simulink model "AuxClimaCtlMgr"  (R2022b)
settings: Solver=FixedStepDiscrete  StartTime=0.0  StopTime=10.0  FixedStep=0.2  RelTol=1e-3
(part 2 of 2)

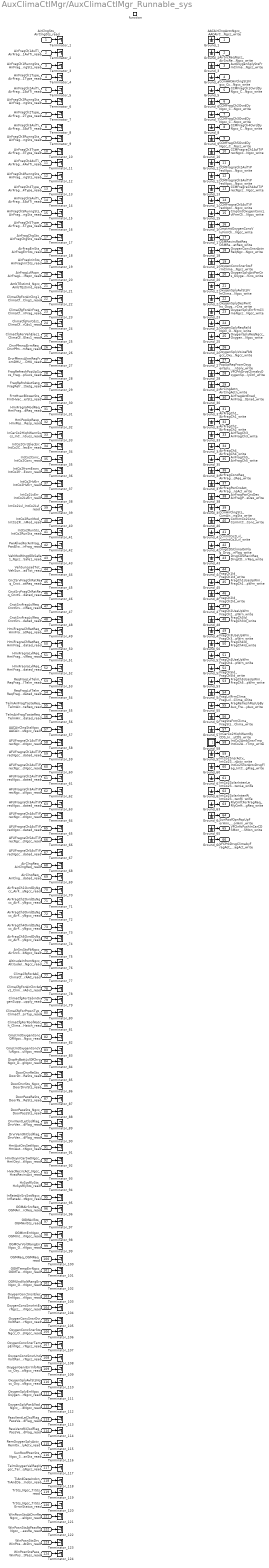
== system AuxClimaCtlMgr/AuxClimaCtlMgr_Runnable_sys ==
  AirClngSts_AirClngSts_read : Inport
  AirFragCh1AvlTi_AirFragCh1AvlTi_read : Inport  Port=2
  AirFragCh1RunngSts_AirFragCh1RunngSts_read : Inport  Port=3
  AirFragCh1Type_AirFragCh1Type_read : Inport  Port=4
  AirFragCh2AvlTi_AirFragCh2AvlTi_read : Inport  Port=5
  AirFragCh2RunngSts_AirFragCh2RunngSts_read : Inport  Port=6
  AirFragCh2Type_AirFragCh2Type_read : Inport  Port=7
  AirFragCh3AvlTi_AirFragCh3AvlTi_read : Inport  Port=8
  AirFragCh3RunngSts_AirFragCh3RunngSts_read : Inport  Port=9
  AirFragCh3Type_AirFragCh3Type_read : Inport  Port=10
  AirFragCh4AvlTi_AirFragCh4AvlTi_read : Inport  Port=11
  AirFragCh4RunngSts_AirFragCh4RunngSts_read : Inport  Port=12
  AirFragCh4Type_AirFragCh4Type_read : Inport  Port=13
  AirFragCh5AvlTi_AirFragCh5AvlTi_read : Inport  Port=14
  AirFragCh5RunngSts_AirFragCh5RunngSts_read : Inport  Port=15
  AirFragCh5Type_AirFragCh5Type_read : Inport  Port=16
  AirFragChgSts_AirFragChgSts_read : Inport  Port=17
  AirFragErrSts_AirFragErrSts_read : Inport  Port=18
  AirFragInitSts_AirFragInitSts_read : Inport  Port=19
  AirFragLvlRspn_AirFragLvlRspn_read : Inport  Port=20
  AmbTEstimd_Ngcc_AmbTEstimd_read : Inport  Port=21
  ClimaCfgForAirClng1_ClimaCfgForAirClng1_read : Inport  Port=22
  ClimaCfgForAirFrag_ClimaCfgForAirFrag_read : Inport  Port=23
  ClimaCfgForCds1_ClimaCfgForCds1_read : Inport  Port=24
  ClimaCfgForVehElec1_ClimaCfgForVehElec1_read : Inport  Port=25
  DrvrPfmncAlrmReq_DrvrPfmncAlrmReq_read : Inport  Port=26
  DrvrPfmncAlrmReqFromDHU_DrvrPfmncAlrmReqFromDHU_read : Inport  Port=27
  FragRefreshPopUpSucs_FragRefreshPopUpSucs_read : Inport  Port=28
  FragRefrshAutSetg_FragRefrshAutSetg_read : Inport  Port=29
  FrntHvacBlowerSts_FrntHvacBlowerSts_read : Inport  Port=30
  HmiFragraModReq_HmiFragraModReq_read : Inport  Port=31
  HmiPopUpResp_HmiPopUpResp_read : Inport  Port=32
  InCarCo2HighWarnSucs_InCarCo2HighWarnSucs_read : Inport  Port=33
  IntCo2CircElecErr_IntCo2CircElecErr_read : Inport  Port=34
  IntCo2Conc_IntCo2Conc_read : Inport  Port=35
  IntCo2fromExsrc_IntCo2fromExsrc_read : Inport  Port=36
  IntCo2HvErr_IntCo2HvErr_read : Inport  Port=37
  IntCo2LvErr_IntCo2LvErr_read : Inport  Port=38
  IntCo2Lvl_IntCo2Lvl_read : Inport  Port=39
  IntCo2RunMod_IntCo2RunMod_read : Inport  Port=40
  IntCo2RunSts_IntCo2RunSts_read : Inport  Port=41
  PwrAllwdForAirFrag_PwrAllwdForAirFrag_read : Inport  Port=42
  VehModMngtGlbSafe1_Ngcc_VehModMngtGlbSafe1_read : Inport  Port=43
  VehSunLoadTot_VehSunLoadTot_read : Inport  Port=44
  CnctSrvFragChRatReq_CnctSrvFragChRatReq_read : Inport  Port=45
  CnctSrvFragChRatReq_CnctSrvFragChRatReq_IsUpdated_read : Inport  Port=46
  CnctSrvFragLvlReq_CnctSrvFragLvlReq_read : Inport  Port=47
  CnctSrvFragLvlReq_CnctSrvFragLvlReq_IsUpdated_read : Inport  Port=48
  HmiFragraChRatReq_HmiFragraChRatReq_read : Inport  Port=49
  HmiFragraChRatReq_HmiFragraChRatReq_IsUpdated_read : Inport  Port=50
  HmiFragraLvlReq_HmiFragraLvlReq_read : Inport  Port=51
  HmiFragraLvlReq_HmiFragraLvlReq_IsUpdated_read : Inport  Port=52
  ReqFragLvlTelm_ReqFragLvlTelm_read : Inport  Port=53
  ReqFragLvlTelm_ReqFragLvlTelm_IsUpdated_read : Inport  Port=54
  TelmAirFragTasteReq_TelmAirFragTasteReq_read : Inport  Port=55
  TelmAirFragTasteReq_TelmAirFragTasteReq_IsUpdated_read : Inport  Port=56
  AACAirClngStsNgcc_AACAirClngStsNgcc_read : Inport  Port=57
  AFUFragraCh1AvlTiPrecNgcc_AFUFragraCh1AvlTiPrecNgcc_read : Inport  Port=58
  AFUFragraCh1AvlTiPrecNgcc_AFUFragraCh1AvlTiPrecNgcc_IsUpdated_read : Inport  Port=59
  AFUFragraCh2AvlTiPrecNgcc_AFUFragraCh2AvlTiPrecNgcc_read : Inport  Port=60
  AFUFragraCh2AvlTiPrecNgcc_AFUFragraCh2AvlTiPrecNgcc_IsUpdated_read : Inport  Port=61
  AFUFragraCh3AvlTiPrecNgcc_AFUFragraCh3AvlTiPrecNgcc_read : Inport  Port=62
  AFUFragraCh3AvlTiPrecNgcc_AFUFragraCh3AvlTiPrecNgcc_IsUpdated_read : Inport  Port=63
  AFUFragraCh4AvlTiPrecNgcc_AFUFragraCh4AvlTiPrecNgcc_read : Inport  Port=64
  AFUFragraCh4AvlTiPrecNgcc_AFUFragraCh4AvlTiPrecNgcc_IsUpdated_read : Inport  Port=65
  AFUFragraCh5AvlTiPrecNgcc_AFUFragraCh5AvlTiPrecNgcc_read : Inport  Port=66
  AFUFragraCh5AvlTiPrecNgcc_AFUFragraCh5AvlTiPrecNgcc_IsUpdated_read : Inport  Port=67
  AirClngReq_AirClngReq_read : Inport  Port=68
  AirClngReq_AirClngReq_IsUpdated_read : Inport  Port=69
  AirFragCh1OvrdDyNgcc_AirFragCh1OvrdDyNgcc_read : Inport  Port=70
  AirFragCh2OvrdDyNgcc_AirFragCh2OvrdDyNgcc_read : Inport  Port=71
  AirFragCh3OvrdDyNgcc_AirFragCh3OvrdDyNgcc_read : Inport  Port=72
  AirFragCh4OvrdDyNgcc_AirFragCh4OvrdDyNgcc_read : Inport  Port=73
  AirFragCh5OvrdDyNgcc_AirFragCh5OvrdDyNgcc_read : Inport  Port=74
  AirSrcStsFbNgcc_AirSrcStsFbNgcc_read : Inport  Port=75
  AltitudeInfromNgcc_AltitudeInfromNgcc_read : Inport  Port=76
  ClimaCfgForAAC_ClimaCfgForAAC_read : Inport  Port=77
  ClimaCfgForAirClnrAdv1_ClimaCfgForAirClnrAdv1_read : Inport  Port=78
  ClimaCfgForCabinOxgenSupply_ClimaCfgForCabinOxgenSupply_read : Inport  Port=79
  ClimaCfgForPrpsnTyp_ClimaCfgForPrpsnTyp_read : Inport  Port=80
  ClimaCfgForRoofHatch_ClimaCfgForRoofHatch_read : Inport  Port=81
  CmptmtOxygenConcQFNgcc_CmptmtOxygenConcQFNgcc_read : Inport  Port=82
  CmptmtOxygenConcVluNgcc_CmptmtOxygenConcVluNgcc_read : Inport  Port=83
  DispHvBattLvlOfChrgNgcc_DispHvBattLvlOfChrgNgcc_read : Inport  Port=84
  DoorDrvrReSts_DoorDrvrReSts_read : Inport  Port=85
  DoorDrvrSts_Ngcc_DoorDrvrSts_read : Inport  Port=86
  DoorPassReSts_DoorPassReSts_read : Inport  Port=87
  DoorPassSts_Ngcc_DoorPassSts_read : Inport  Port=88
  DrvrVentLeClsdFlag_DrvrVentLeClsdFlag_read : Inport  Port=89
  DrvrVentRiClsdFlag_DrvrVentRiClsdFlag_read : Inport  Port=90
  HmiAutOxySetNgcc_HmiAutOxySetNgcc_read : Inport  Port=91
  HmiOxyinCarSwtNgcc_HmiOxyinCarSwtNgcc_read : Inport  Port=92
  HvacRecircAct_Ngcc_HvacRecircAct_read : Inport  Port=93
  HvSysRlySts_HvSysRlySts_read : Inport  Port=94
  InflateAirSrcSwtNgcc_InflateAirSrcSwtNgcc_read : Inport  Port=95
  OGMAirSrcReq_OGMAirSrcReq_read : Inport  Port=96
  OGMAvlSts_OGMAvlSts_read : Inport  Port=97
  OGMIntErrNgcc_OGMIntErrNgcc_read : Inport  Port=98
  OGMOvrVoltRangErrNgcc_OGMOvrVoltRangErrNgcc_read : Inport  Port=99
  OGMReq_OGMReq_read : Inport  Port=100
  OGMTempErrNgcc_OGMTempErrNgcc_read : Inport  Port=101
  OGMUndVoltRangErrNgcc_OGMUndVoltRangErrNgcc_read : Inport  Port=102
  OxygenConcSnsrElecErrNgcc_OxygenConcSnsrElecErrNgcc_read : Inport  Port=103
  OxygenConcSnsrIntErrNgcc_OxygenConcSnsrIntErrNgcc_read : Inport  Port=104
  OxygenConcSnsrOvrVoltRangErrNgcc_OxygenConcSnsrOvrVoltRangErrNgcc_read : Inport  Port=105
  OxygenConcSnsrStsNgcc_OxygenConcSnsrStsNgcc_read : Inport  Port=106
  OxygenConcSnsrTempErrNgcc_OxygenConcSnsrTempErrNgcc_read : Inport  Port=107
  OxygenConcSnsrUndVoltRangErrNgcc_OxygenConcSnsrUndVoltRangErrNgcc_read : Inport  Port=108
  OxygenGenrErrInfoNgcc_OxygenGenrErrInfoNgcc_read : Inport  Port=109
  OxygenSplyAvlStsNgcc_OxygenSplyAvlStsNgcc_read : Inport  Port=110
  OxygenSplyErrNgcc_OxygenSplyErrNgcc_read : Inport  Port=111
  OxygenSplyPwrAllwdNgcc_OxygenSplyPwrAllwdNgcc_read : Inport  Port=112
  PassVentLeClsdFlag_PassVentLeClsdFlag_read : Inport  Port=113
  PassVentRiClsdFlag_PassVentRiClsdFlag_read : Inport  Port=114
  RemOxygenSplyActv_RemOxygenSplyActv_read : Inport  Port=115
  SunRoofPosnSts_Ngcc_SunRoofPosnSts_read : Inport  Port=116
  TelmOxygenValReqNgcc_TelmOxygenValReqNgcc_read : Inport  Port=117
  TiAndDateIndcn_TiAndDateIndcn_read : Inport  Port=118
  TrSts_Ngcc_TrSts_read : Inport  Port=119
  TrSts_Ngcc_TrSts_ErrorStatus_read : Inport  Port=120
  WinPosnStsAtDrvrReNgcc_WinPosnStsAtDrvrReNgcc_read : Inport  Port=121
  WinPosnStsAtPassReNgcc_WinPosnStsAtPassRe_read : Inport  Port=122
  WinPosnStsDrv_WinPosnStsAtDrv_read : Inport  Port=123
  WinPosnStsPass_WinPosnStsAtPass_read : Inport  Port=124
  function : TriggerPort  TriggerType=function-call  VariantControl=(inherit)  SampleTimeType=periodic  SampleTime=-1
  Ground_{1..57,59..66} (65 alike) : Ground
  Ground_58 : Subsystem
  Terminator_{1..124} (124 alike) : Terminator
  AACAirClngActnNgcc_AACAirClngActnNgcc_write : Outport
      settings: EnsureOutportIsVirtual=on
  AirSrcReqNgcc_AirSrcReqNgcc_write : Outport  Port=2
      settings: EnsureOutportIsVirtual=on
  AutOxygenSplyStsFrmClimaNgcc_AutOxygenSplyStsFrmClimaNgcc_write : Outport  Port=3
      settings: EnsureOutportIsVirtual=on
  CcmAACAirClngStsNgcc_CcmAACAirClngStsNgcc_write : Outport  Port=4
      settings: EnsureOutportIsVirtual=on
  CCMFragCh1OvrdDyNgcc_CCMFragCh1OvrdDyNgcc_write : Outport  Port=5
      settings: EnsureOutportIsVirtual=on
  CCMFragCh2OvrdDyNgcc_CCMFragCh2OvrdDyNgcc_write : Outport  Port=6
      settings: EnsureOutportIsVirtual=on
  CCMFragCh3OvrdDyNgcc_CCMFragCh3OvrdDyNgcc_write : Outport  Port=7
      settings: EnsureOutportIsVirtual=on
  CCMFragCh4OvrdDyNgcc_CCMFragCh4OvrdDyNgcc_write : Outport  Port=8
      settings: EnsureOutportIsVirtual=on
  CCMFragCh5OvrdDyNgcc_CCMFragCh5OvrdDyNgcc_write : Outport  Port=9
      settings: EnsureOutportIsVirtual=on
  CCMFragraCh1AvlTiPrecNgcc_CCMFragraCh1AvlTiPrecNgcc_write : Outport  Port=10
      settings: EnsureOutportIsVirtual=on
  CCMFragraCh2AvlTiPrecNgcc_CCMFragraCh2AvlTiPrecNgcc_write : Outport  Port=11
      settings: EnsureOutportIsVirtual=on
  CCMFragraCh3AvlTiPrecNgcc_CCMFragraCh3AvlTiPrecNgcc_write : Outport  Port=12
      settings: EnsureOutportIsVirtual=on
  CCMFragraCh4AvlTiPrecNgcc_CCMFragraCh4AvlTiPrecNgcc_write : Outport  Port=13
      settings: EnsureOutportIsVirtual=on
  CCMFragraCh5AvlTiPrecNgcc_CCMFragraCh5AvlTiPrecNgcc_write : Outport  Port=14
      settings: EnsureOutportIsVirtual=on
  CmptmtOxygenConcLvlFrmClimaNgcc_CmptmtOxygenConcLvlFrmClimaNgcc_write : Outport  Port=15
      settings: EnsureOutportIsVirtual=on
  CmptmtOxygenConcVluFrmClimaNgcc_CmptmtOxygenConcVluFrmClimaNgcc_write : Outport  Port=16
      settings: EnsureOutportIsVirtual=on
  OSMRecircRatReq_OSMRecircRatReq_write : Outport  Port=17
      settings: EnsureOutportIsVirtual=on
  OxygenConcSnsrActnReqNgcc_OxygenConcSnsrActnReqNgcc_write : Outport  Port=18
      settings: EnsureOutportIsVirtual=on
  OxygenConcSnsrStsFrmClimaNgcc_OxygenConcSnsrStsFrmClimaNgcc_write : Outport  Port=19
      settings: EnsureOutportIsVirtual=on
  OxygenSplyActPwrCns_OxygenSplyActPwrCns_write : Outport  Port=20
      settings: EnsureOutportIsVirtual=on
  OxygenSplyAvlStsFrmClimaNgcc_OxygenSplyAvlStsFrmClimaNgcc_write : Outport  Port=21
      settings: EnsureOutportIsVirtual=on
  OxygenSplyDesPwrCns_OxygenSplyDesPwrCns_write : Outport  Port=22
      settings: EnsureOutportIsVirtual=on
  OxygenSplyErrFrmClimaNgcc_OxygenSplyErrFrmClimaNgcc_write : Outport  Port=23
      settings: EnsureOutportIsVirtual=on
  OxygenSplyReqFaildNgcc_OxygenSplyReqFaildNgcc_write : Outport  Port=24
      settings: EnsureOutportIsVirtual=on
  OxygenSplyReqNgcc_OxygenSplyReqNgcc_write : Outport  Port=25
      settings: EnsureOutportIsVirtual=on
  OxygenSplyVoiceFbNgcc_OxygenSplyVoiceFbNgcc_write : Outport  Port=26
      settings: EnsureOutportIsVirtual=on
  PopUpReqFromOxygenSply_PopUpReqFromOxygenSply_write : Outport  Port=27
      settings: EnsureOutportIsVirtual=on
  VFCPrkDrvgClimabyOxygenSplyCtrl_VFCPrkDrvgClimabyOxygenSplyCtrl_write : Outport  Port=28
      settings: EnsureOutportIsVirtual=on
  AirClngActn_AirClngActn_write : Outport  Port=29
      settings: EnsureOutportIsVirtual=on
  AirFragActEnad_AirFragActEnad_write : Outport  Port=30
      settings: EnsureOutportIsVirtual=on
  AirFragCh1_AirFragCh1_write : Outport  Port=31
      settings: EnsureOutportIsVirtual=on
  AirFragCh2_AirFragCh2_write : Outport  Port=32
      settings: EnsureOutportIsVirtual=on
  AirFragCh3_AirFragCh3_write : Outport  Port=33
      settings: EnsureOutportIsVirtual=on
  AirFragCh4_AirFragCh4_write : Outport  Port=34
      settings: EnsureOutportIsVirtual=on
  AirFragCh5_AirFragCh5_write : Outport  Port=35
      settings: EnsureOutportIsVirtual=on
  AirFragConcReq_AirFragConcReq_write : Outport  Port=36
      settings: EnsureOutportIsVirtual=on
  AirFragPwrCnsAct_AirFragPwrCnsAct_write : Outport  Port=37
      settings: EnsureOutportIsVirtual=on
  AirFragPwrCnsDes_AirFragPwrCnsDes_write : Outport  Port=38
      settings: EnsureOutportIsVirtual=on
  CcmAirClngSts_CcmAirClngSts_write : Outport  Port=39
      settings: EnsureOutportIsVirtual=on
  CcmIntCo2Conc_CcmIntCo2Conc_write : Outport  Port=40
      settings: EnsureOutportIsVirtual=on
  CcmIntCo2Lvl_CcmIntCo2Lvl_write : Outport  Port=41
      settings: EnsureOutportIsVirtual=on
  DrvgCDSClimaOnFlag_DrvgCDSClimaOnFlag_write : Outport  Port=42
      settings: EnsureOutportIsVirtual=on
  DrvgCDSRecirReq_DrvgCDSRecirReq_write : Outport  Port=43
      settings: EnsureOutportIsVirtual=on
  FragCh1Id_FragCh1Id_write : Outport  Port=44
      settings: EnsureOutportIsVirtual=on
  FragCh1UseUpWrn_FragCh1UseUpWrn_write : Outport  Port=45
      settings: EnsureOutportIsVirtual=on
  FragCh2Id_FragCh2Id_write : Outport  Port=46
      settings: EnsureOutportIsVirtual=on
  FragCh2UseUpWrn_FragCh2UseUpWrn_write : Outport  Port=47
      settings: EnsureOutportIsVirtual=on
  FragCh3Id_FragCh3Id_write : Outport  Port=48
      settings: EnsureOutportIsVirtual=on
  FragCh3UseUpWrn_FragCh3UseUpWrn_write : Outport  Port=49
      settings: EnsureOutportIsVirtual=on
  FragCh4Id_FragCh4Id_write : Outport  Port=50
      settings: EnsureOutportIsVirtual=on
  FragCh4UseUpWrn_FragCh4UseUpWrn_write : Outport  Port=51
      settings: EnsureOutportIsVirtual=on
  FragCh5Id_FragCh5Id_write : Outport  Port=52
      settings: EnsureOutportIsVirtual=on
  FragCh5UseUpWrn_FragCh5UseUpWrn_write : Outport  Port=53
      settings: EnsureOutportIsVirtual=on
  FragLvlFrmClima_FragLvlFrmClima_write : Outport  Port=54
      settings: EnsureOutportIsVirtual=on
  FragRefreshPopUpByAux_FragRefreshPopUpByAux_write : Outport  Port=55
      settings: EnsureOutportIsVirtual=on
  FragStsFrmClima_FragStsFrmClima_write : Outport  Port=56
      settings: EnsureOutportIsVirtual=on
  InCarCo2HighWarnByCDS_InCarCo2HighWarnByCDS_write : Outport  Port=57
      settings: EnsureOutportIsVirtual=on
  IntCo2AmbSnsrTmp_IntCo2AmbSnsrTmp_write : Outport  Port=58
      settings: EnsureOutportIsVirtual=on
  IntCo2SnsrActv_IntCo2SnsrActv_write : Outport  Port=59
      settings: EnsureOutportIsVirtual=on
  IntCo2SnsrActvDrvgFlag_IntCo2SnsrActvDrvgFlag_write : Outport  Port=60
      settings: EnsureOutportIsVirtual=on
  IntCo2SolarIntenLe_IntCo2SolarIntenLe_write : Outport  Port=61
      settings: EnsureOutportIsVirtual=on
  IntCo2SolarIntenRi_IntCo2SolarIntenRi_write : Outport  Port=62
      settings: EnsureOutportIsVirtual=on
  RlyCmftForFragReq_RlyCmftForFragReq_write : Outport  Port=63
      settings: EnsureOutportIsVirtual=on
  SunRoofOpnPopUpForHmi_SunRoofOpnPopUpForHmi_write : Outport  Port=64
      settings: EnsureOutportIsVirtual=on
  VFCInfoPushInCarCDSMon_VFCInfoPushInCarCDSMon_write : Outport  Port=65
      settings: EnsureOutportIsVirtual=on
  VFCPrkDrvgClimabyFragAct_VFCPrkDrvgClimabyFragAct_write : Outport  Port=66
      settings: EnsureOutportIsVirtual=on
  wires:
  AirClngSts_AirClngSts_read/1 -> Terminator_1/1
  AirFragCh1AvlTi_AirFragCh1AvlTi_read/1 -> Terminator_2/1
  AirFragCh1RunngSts_AirFragCh1RunngSts_read/1 -> Terminator_3/1
  AirFragCh1Type_AirFragCh1Type_read/1 -> Terminator_4/1
  AirFragCh2AvlTi_AirFragCh2AvlTi_read/1 -> Terminator_5/1
  AirFragCh2RunngSts_AirFragCh2RunngSts_read/1 -> Terminator_6/1
  AirFragCh2Type_AirFragCh2Type_read/1 -> Terminator_7/1
  AirFragCh3AvlTi_AirFragCh3AvlTi_read/1 -> Terminator_8/1
  AirFragCh3RunngSts_AirFragCh3RunngSts_read/1 -> Terminator_9/1
  AirFragCh3Type_AirFragCh3Type_read/1 -> Terminator_10/1
  AirFragCh4AvlTi_AirFragCh4AvlTi_read/1 -> Terminator_11/1
  AirFragCh4RunngSts_AirFragCh4RunngSts_read/1 -> Terminator_12/1
  AirFragCh4Type_AirFragCh4Type_read/1 -> Terminator_13/1
  AirFragCh5AvlTi_AirFragCh5AvlTi_read/1 -> Terminator_14/1
  AirFragCh5RunngSts_AirFragCh5RunngSts_read/1 -> Terminator_15/1
  AirFragCh5Type_AirFragCh5Type_read/1 -> Terminator_16/1
  AirFragChgSts_AirFragChgSts_read/1 -> Terminator_17/1
  AirFragErrSts_AirFragErrSts_read/1 -> Terminator_18/1
  AirFragInitSts_AirFragInitSts_read/1 -> Terminator_19/1
  AirFragLvlRspn_AirFragLvlRspn_read/1 -> Terminator_20/1
  AmbTEstimd_Ngcc_AmbTEstimd_read/1 -> Terminator_21/1
  ClimaCfgForAirClng1_ClimaCfgForAirClng1_read/1 -> Terminator_22/1
  ClimaCfgForAirFrag_ClimaCfgForAirFrag_read/1 -> Terminator_23/1
  ClimaCfgForCds1_ClimaCfgForCds1_read/1 -> Terminator_24/1
  ClimaCfgForVehElec1_ClimaCfgForVehElec1_read/1 -> Terminator_25/1
  DrvrPfmncAlrmReq_DrvrPfmncAlrmReq_read/1 -> Terminator_26/1
  DrvrPfmncAlrmReqFromDHU_DrvrPfmncAlrmReqFromDHU_read/1 -> Terminator_27/1
  FragRefreshPopUpSucs_FragRefreshPopUpSucs_read/1 -> Terminator_28/1
  FragRefrshAutSetg_FragRefrshAutSetg_read/1 -> Terminator_29/1
  FrntHvacBlowerSts_FrntHvacBlowerSts_read/1 -> Terminator_30/1
  HmiFragraModReq_HmiFragraModReq_read/1 -> Terminator_31/1
  HmiPopUpResp_HmiPopUpResp_read/1 -> Terminator_32/1
  InCarCo2HighWarnSucs_InCarCo2HighWarnSucs_read/1 -> Terminator_33/1
  IntCo2CircElecErr_IntCo2CircElecErr_read/1 -> Terminator_34/1
  IntCo2Conc_IntCo2Conc_read/1 -> Terminator_35/1
  IntCo2fromExsrc_IntCo2fromExsrc_read/1 -> Terminator_36/1
  IntCo2HvErr_IntCo2HvErr_read/1 -> Terminator_37/1
  IntCo2LvErr_IntCo2LvErr_read/1 -> Terminator_38/1
  IntCo2Lvl_IntCo2Lvl_read/1 -> Terminator_39/1
  IntCo2RunMod_IntCo2RunMod_read/1 -> Terminator_40/1
  IntCo2RunSts_IntCo2RunSts_read/1 -> Terminator_41/1
  PwrAllwdForAirFrag_PwrAllwdForAirFrag_read/1 -> Terminator_42/1
  VehModMngtGlbSafe1_Ngcc_VehModMngtGlbSafe1_read/1 -> Terminator_43/1
  VehSunLoadTot_VehSunLoadTot_read/1 -> Terminator_44/1
  CnctSrvFragChRatReq_CnctSrvFragChRatReq_read/1 -> Terminator_45/1
  CnctSrvFragChRatReq_CnctSrvFragChRatReq_IsUpdated_read/1 -> Terminator_46/1
  CnctSrvFragLvlReq_CnctSrvFragLvlReq_read/1 -> Terminator_47/1
  CnctSrvFragLvlReq_CnctSrvFragLvlReq_IsUpdated_read/1 -> Terminator_48/1
  HmiFragraChRatReq_HmiFragraChRatReq_read/1 -> Terminator_49/1
  HmiFragraChRatReq_HmiFragraChRatReq_IsUpdated_read/1 -> Terminator_50/1
  HmiFragraLvlReq_HmiFragraLvlReq_read/1 -> Terminator_51/1
  HmiFragraLvlReq_HmiFragraLvlReq_IsUpdated_read/1 -> Terminator_52/1
  ReqFragLvlTelm_ReqFragLvlTelm_read/1 -> Terminator_53/1
  ReqFragLvlTelm_ReqFragLvlTelm_IsUpdated_read/1 -> Terminator_54/1
  TelmAirFragTasteReq_TelmAirFragTasteReq_read/1 -> Terminator_55/1
  TelmAirFragTasteReq_TelmAirFragTasteReq_IsUpdated_read/1 -> Terminator_56/1
  AACAirClngStsNgcc_AACAirClngStsNgcc_read/1 -> Terminator_57/1
  AFUFragraCh1AvlTiPrecNgcc_AFUFragraCh1AvlTiPrecNgcc_read/1 -> Terminator_58/1
  AFUFragraCh1AvlTiPrecNgcc_AFUFragraCh1AvlTiPrecNgcc_IsUpdated_read/1 -> Terminator_59/1
  AFUFragraCh2AvlTiPrecNgcc_AFUFragraCh2AvlTiPrecNgcc_read/1 -> Terminator_60/1
  AFUFragraCh2AvlTiPrecNgcc_AFUFragraCh2AvlTiPrecNgcc_IsUpdated_read/1 -> Terminator_61/1
  AFUFragraCh3AvlTiPrecNgcc_AFUFragraCh3AvlTiPrecNgcc_read/1 -> Terminator_62/1
  AFUFragraCh3AvlTiPrecNgcc_AFUFragraCh3AvlTiPrecNgcc_IsUpdated_read/1 -> Terminator_63/1
  AFUFragraCh4AvlTiPrecNgcc_AFUFragraCh4AvlTiPrecNgcc_read/1 -> Terminator_64/1
  AFUFragraCh4AvlTiPrecNgcc_AFUFragraCh4AvlTiPrecNgcc_IsUpdated_read/1 -> Terminator_65/1
  AFUFragraCh5AvlTiPrecNgcc_AFUFragraCh5AvlTiPrecNgcc_read/1 -> Terminator_66/1
  AFUFragraCh5AvlTiPrecNgcc_AFUFragraCh5AvlTiPrecNgcc_IsUpdated_read/1 -> Terminator_67/1
  AirClngReq_AirClngReq_read/1 -> Terminator_68/1
  AirClngReq_AirClngReq_IsUpdated_read/1 -> Terminator_69/1
  AirFragCh1OvrdDyNgcc_AirFragCh1OvrdDyNgcc_read/1 -> Terminator_70/1
  AirFragCh2OvrdDyNgcc_AirFragCh2OvrdDyNgcc_read/1 -> Terminator_71/1
  AirFragCh3OvrdDyNgcc_AirFragCh3OvrdDyNgcc_read/1 -> Terminator_72/1
  AirFragCh4OvrdDyNgcc_AirFragCh4OvrdDyNgcc_read/1 -> Terminator_73/1
  AirFragCh5OvrdDyNgcc_AirFragCh5OvrdDyNgcc_read/1 -> Terminator_74/1
  AirSrcStsFbNgcc_AirSrcStsFbNgcc_read/1 -> Terminator_75/1
  AltitudeInfromNgcc_AltitudeInfromNgcc_read/1 -> Terminator_76/1
  ClimaCfgForAAC_ClimaCfgForAAC_read/1 -> Terminator_77/1
  ClimaCfgForAirClnrAdv1_ClimaCfgForAirClnrAdv1_read/1 -> Terminator_78/1
  ClimaCfgForCabinOxgenSupply_ClimaCfgForCabinOxgenSupply_read/1 -> Terminator_79/1
  ClimaCfgForPrpsnTyp_ClimaCfgForPrpsnTyp_read/1 -> Terminator_80/1
  ClimaCfgForRoofHatch_ClimaCfgForRoofHatch_read/1 -> Terminator_81/1
  CmptmtOxygenConcQFNgcc_CmptmtOxygenConcQFNgcc_read/1 -> Terminator_82/1
  CmptmtOxygenConcVluNgcc_CmptmtOxygenConcVluNgcc_read/1 -> Terminator_83/1
  DispHvBattLvlOfChrgNgcc_DispHvBattLvlOfChrgNgcc_read/1 -> Terminator_84/1
  DoorDrvrReSts_DoorDrvrReSts_read/1 -> Terminator_85/1
  DoorDrvrSts_Ngcc_DoorDrvrSts_read/1 -> Terminator_86/1
  DoorPassReSts_DoorPassReSts_read/1 -> Terminator_87/1
  DoorPassSts_Ngcc_DoorPassSts_read/1 -> Terminator_88/1
  DrvrVentLeClsdFlag_DrvrVentLeClsdFlag_read/1 -> Terminator_89/1
  DrvrVentRiClsdFlag_DrvrVentRiClsdFlag_read/1 -> Terminator_90/1
  HmiAutOxySetNgcc_HmiAutOxySetNgcc_read/1 -> Terminator_91/1
  HmiOxyinCarSwtNgcc_HmiOxyinCarSwtNgcc_read/1 -> Terminator_92/1
  HvacRecircAct_Ngcc_HvacRecircAct_read/1 -> Terminator_93/1
  HvSysRlySts_HvSysRlySts_read/1 -> Terminator_94/1
  InflateAirSrcSwtNgcc_InflateAirSrcSwtNgcc_read/1 -> Terminator_95/1
  OGMAirSrcReq_OGMAirSrcReq_read/1 -> Terminator_96/1
  OGMAvlSts_OGMAvlSts_read/1 -> Terminator_97/1
  OGMIntErrNgcc_OGMIntErrNgcc_read/1 -> Terminator_98/1
  OGMOvrVoltRangErrNgcc_OGMOvrVoltRangErrNgcc_read/1 -> Terminator_99/1
  OGMReq_OGMReq_read/1 -> Terminator_100/1
  OGMTempErrNgcc_OGMTempErrNgcc_read/1 -> Terminator_101/1
  OGMUndVoltRangErrNgcc_OGMUndVoltRangErrNgcc_read/1 -> Terminator_102/1
  OxygenConcSnsrElecErrNgcc_OxygenConcSnsrElecErrNgcc_read/1 -> Terminator_103/1
  OxygenConcSnsrIntErrNgcc_OxygenConcSnsrIntErrNgcc_read/1 -> Terminator_104/1
  OxygenConcSnsrOvrVoltRangErrNgcc_OxygenConcSnsrOvrVoltRangErrNgcc_read/1 -> Terminator_105/1
  OxygenConcSnsrStsNgcc_OxygenConcSnsrStsNgcc_read/1 -> Terminator_106/1
  OxygenConcSnsrTempErrNgcc_OxygenConcSnsrTempErrNgcc_read/1 -> Terminator_107/1
  OxygenConcSnsrUndVoltRangErrNgcc_OxygenConcSnsrUndVoltRangErrNgcc_read/1 -> Terminator_108/1
  OxygenGenrErrInfoNgcc_OxygenGenrErrInfoNgcc_read/1 -> Terminator_109/1
  OxygenSplyAvlStsNgcc_OxygenSplyAvlStsNgcc_read/1 -> Terminator_110/1
  OxygenSplyErrNgcc_OxygenSplyErrNgcc_read/1 -> Terminator_111/1
  OxygenSplyPwrAllwdNgcc_OxygenSplyPwrAllwdNgcc_read/1 -> Terminator_112/1
  PassVentLeClsdFlag_PassVentLeClsdFlag_read/1 -> Terminator_113/1
  PassVentRiClsdFlag_PassVentRiClsdFlag_read/1 -> Terminator_114/1
  RemOxygenSplyActv_RemOxygenSplyActv_read/1 -> Terminator_115/1
  SunRoofPosnSts_Ngcc_SunRoofPosnSts_read/1 -> Terminator_116/1
  TelmOxygenValReqNgcc_TelmOxygenValReqNgcc_read/1 -> Terminator_117/1
  TiAndDateIndcn_TiAndDateIndcn_read/1 -> Terminator_118/1
  TrSts_Ngcc_TrSts_read/1 -> Terminator_119/1
  TrSts_Ngcc_TrSts_ErrorStatus_read/1 -> Terminator_120/1
  WinPosnStsAtDrvrReNgcc_WinPosnStsAtDrvrReNgcc_read/1 -> Terminator_121/1
  WinPosnStsAtPassReNgcc_WinPosnStsAtPassRe_read/1 -> Terminator_122/1
  WinPosnStsDrv_WinPosnStsAtDrv_read/1 -> Terminator_123/1
  WinPosnStsPass_WinPosnStsAtPass_read/1 -> Terminator_124/1
  Ground_1/1 -> AACAirClngActnNgcc_AACAirClngActnNgcc_write/1
  Ground_2/1 -> AirSrcReqNgcc_AirSrcReqNgcc_write/1
  Ground_3/1 -> AutOxygenSplyStsFrmClimaNgcc_AutOxygenSplyStsFrmClimaNgcc_write/1
  Ground_4/1 -> CcmAACAirClngStsNgcc_CcmAACAirClngStsNgcc_write/1
  Ground_5/1 -> CCMFragCh1OvrdDyNgcc_CCMFragCh1OvrdDyNgcc_write/1
  Ground_6/1 -> CCMFragCh2OvrdDyNgcc_CCMFragCh2OvrdDyNgcc_write/1
  Ground_7/1 -> CCMFragCh3OvrdDyNgcc_CCMFragCh3OvrdDyNgcc_write/1
  Ground_8/1 -> CCMFragCh4OvrdDyNgcc_CCMFragCh4OvrdDyNgcc_write/1
  Ground_9/1 -> CCMFragCh5OvrdDyNgcc_CCMFragCh5OvrdDyNgcc_write/1
  Ground_10/1 -> CCMFragraCh1AvlTiPrecNgcc_CCMFragraCh1AvlTiPrecNgcc_write/1
  Ground_11/1 -> CCMFragraCh2AvlTiPrecNgcc_CCMFragraCh2AvlTiPrecNgcc_write/1
  Ground_12/1 -> CCMFragraCh3AvlTiPrecNgcc_CCMFragraCh3AvlTiPrecNgcc_write/1
  Ground_13/1 -> CCMFragraCh4AvlTiPrecNgcc_CCMFragraCh4AvlTiPrecNgcc_write/1
  Ground_14/1 -> CCMFragraCh5AvlTiPrecNgcc_CCMFragraCh5AvlTiPrecNgcc_write/1
  Ground_15/1 -> CmptmtOxygenConcLvlFrmClimaNgcc_CmptmtOxygenConcLvlFrmClimaNgcc_write/1
  Ground_16/1 -> CmptmtOxygenConcVluFrmClimaNgcc_CmptmtOxygenConcVluFrmClimaNgcc_write/1
  Ground_17/1 -> OSMRecircRatReq_OSMRecircRatReq_write/1
  Ground_18/1 -> OxygenConcSnsrActnReqNgcc_OxygenConcSnsrActnReqNgcc_write/1
  Ground_19/1 -> OxygenConcSnsrStsFrmClimaNgcc_OxygenConcSnsrStsFrmClimaNgcc_write/1
  Ground_20/1 -> OxygenSplyActPwrCns_OxygenSplyActPwrCns_write/1
  Ground_21/1 -> OxygenSplyAvlStsFrmClimaNgcc_OxygenSplyAvlStsFrmClimaNgcc_write/1
  Ground_22/1 -> OxygenSplyDesPwrCns_OxygenSplyDesPwrCns_write/1
  Ground_23/1 -> OxygenSplyErrFrmClimaNgcc_OxygenSplyErrFrmClimaNgcc_write/1
  Ground_24/1 -> OxygenSplyReqFaildNgcc_OxygenSplyReqFaildNgcc_write/1
  Ground_25/1 -> OxygenSplyReqNgcc_OxygenSplyReqNgcc_write/1
  Ground_26/1 -> OxygenSplyVoiceFbNgcc_OxygenSplyVoiceFbNgcc_write/1
  Ground_27/1 -> PopUpReqFromOxygenSply_PopUpReqFromOxygenSply_write/1
  Ground_28/1 -> VFCPrkDrvgClimabyOxygenSplyCtrl_VFCPrkDrvgClimabyOxygenSplyCtrl_write/1
  Ground_29/1 -> AirClngActn_AirClngActn_write/1
  Ground_30/1 -> AirFragActEnad_AirFragActEnad_write/1
  Ground_31/1 -> AirFragCh1_AirFragCh1_write/1
  Ground_32/1 -> AirFragCh2_AirFragCh2_write/1
  Ground_33/1 -> AirFragCh3_AirFragCh3_write/1
  Ground_34/1 -> AirFragCh4_AirFragCh4_write/1
  Ground_35/1 -> AirFragCh5_AirFragCh5_write/1
  Ground_36/1 -> AirFragConcReq_AirFragConcReq_write/1
  Ground_37/1 -> AirFragPwrCnsAct_AirFragPwrCnsAct_write/1
  Ground_38/1 -> AirFragPwrCnsDes_AirFragPwrCnsDes_write/1
  Ground_39/1 -> CcmAirClngSts_CcmAirClngSts_write/1
  Ground_40/1 -> CcmIntCo2Conc_CcmIntCo2Conc_write/1
  Ground_41/1 -> CcmIntCo2Lvl_CcmIntCo2Lvl_write/1
  Ground_42/1 -> DrvgCDSClimaOnFlag_DrvgCDSClimaOnFlag_write/1
  Ground_43/1 -> DrvgCDSRecirReq_DrvgCDSRecirReq_write/1
  Ground_44/1 -> FragCh1Id_FragCh1Id_write/1
  Ground_45/1 -> FragCh1UseUpWrn_FragCh1UseUpWrn_write/1
  Ground_46/1 -> FragCh2Id_FragCh2Id_write/1
  Ground_47/1 -> FragCh2UseUpWrn_FragCh2UseUpWrn_write/1
  Ground_48/1 -> FragCh3Id_FragCh3Id_write/1
  Ground_49/1 -> FragCh3UseUpWrn_FragCh3UseUpWrn_write/1
  Ground_50/1 -> FragCh4Id_FragCh4Id_write/1
  Ground_51/1 -> FragCh4UseUpWrn_FragCh4UseUpWrn_write/1
  Ground_52/1 -> FragCh5Id_FragCh5Id_write/1
  Ground_53/1 -> FragCh5UseUpWrn_FragCh5UseUpWrn_write/1
  Ground_54/1 -> FragLvlFrmClima_FragLvlFrmClima_write/1
  Ground_55/1 -> FragRefreshPopUpByAux_FragRefreshPopUpByAux_write/1
  Ground_56/1 -> FragStsFrmClima_FragStsFrmClima_write/1
  Ground_57/1 -> InCarCo2HighWarnByCDS_InCarCo2HighWarnByCDS_write/1
  Ground_58/1(Out1) -> IntCo2AmbSnsrTmp_IntCo2AmbSnsrTmp_write/1
  Ground_59/1 -> IntCo2SnsrActv_IntCo2SnsrActv_write/1
  Ground_60/1 -> IntCo2SnsrActvDrvgFlag_IntCo2SnsrActvDrvgFlag_write/1
  Ground_61/1 -> IntCo2SolarIntenLe_IntCo2SolarIntenLe_write/1
  Ground_62/1 -> IntCo2SolarIntenRi_IntCo2SolarIntenRi_write/1
  Ground_63/1 -> RlyCmftForFragReq_RlyCmftForFragReq_write/1
  Ground_64/1 -> SunRoofOpnPopUpForHmi_SunRoofOpnPopUpForHmi_write/1
  Ground_65/1 -> VFCInfoPushInCarCDSMon_VFCInfoPushInCarCDSMon_write/1
  Ground_66/1 -> VFCPrkDrvgClimabyFragAct_VFCPrkDrvgClimabyFragAct_write/1

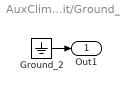
== system AuxClimaCtlMgr/AuxClimaCtlMgr_Init/Ground_2 ==
  Ground_2 : Ground
  Out1 : Outport  OutDataTypeStr=Bus: AmbTEstimd  PortDimensions=1
  wires:
  Ground_2/1 -> Out1/1

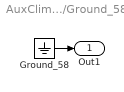
== system AuxClimaCtlMgr/AuxClimaCtlMgr_Runnable_sys/Ground_58 ==
  Ground_58 : Ground
  Out1 : Outport  OutDataTypeStr=Bus: AmbTEstimd  PortDimensions=1
  wires:
  Ground_58/1 -> Out1/1
